simulink model "MX_Gateway"  (Simulink 9.0) (continued)
  CD_FAST_TRAVEL_FUNCTION = Bobcat.Parameter; CreateAliasCD( CD_FAST_TRAVEL_FUNCTION                              ,uint8(255)                 ,'ConstantDataPointer' ,'CONSTANT_DATA' ,'FAST_TRAVEL_FUNCTION')    [cdata.m]    (also in hc12_cdata.m; m4_cdata.m)
  CD_FAST_TRAVEL_THROTTLE_DELAY = Bobcat.Parameter; CreateAliasCD( CD_FAST_TRAVEL_THROTTLE_DELAY                        ,uint16(10)                 ,'ConstantDataPointer' ,'CONSTANT_DATA' ,'FAST_TRAVEL_THROTTLE_DELAY')    [cdata.m]    (also in hc12_cdata.m; m4_cdata.m)
  CD_FAST_TRAVEL_THROTTLE_LIMIT = Bobcat.Parameter; CreateAliasCD( CD_FAST_TRAVEL_THROTTLE_LIMIT                        ,uint16(1000)               ,'ConstantDataPointer' ,'CONSTANT_DATA' ,'FAST_TRAVEL_THROTTLE_LIMIT')    [cdata.m]    (also in hc12_cdata.m; m4_cdata.m)
  CD_FLYWHEEL_TEETH = Bobcat.Parameter; CreateAliasCD( CD_FLYWHEEL_TEETH                                    ,uint8(83)                  ,'ConstantDataPointer' ,'CONSTANT_DATA' ,'FLYWHEEL_TEETH')    [cdata.m]    (also in hc12_cdata.m; m4_cdata.m)
  CD_FUEL_LEVEL_FILTER_FAST_VALUE = Bobcat.Parameter; CreateAliasCD( CD_FUEL_LEVEL_FILTER_FAST_VALUE                      ,uint8(5)                   ,'ConstantDataPointer' ,'CONSTANT_DATA' ,'FUEL_LEVEL_FILTER_FAST_VALUE')    [cdata.m]    (also in hc12_cdata.m; m4_cdata.m)
  CD_FUEL_LEVEL_FILTER_SLOW_VALUE = Bobcat.Parameter; CreateAliasCD( CD_FUEL_LEVEL_FILTER_SLOW_VALUE                      ,uint8(10)                  ,'ConstantDataPointer' ,'CONSTANT_DATA' ,'FUEL_LEVEL_FILTER_SLOW_VALUE')    [cdata.m]    (also in hc12_cdata.m; m4_cdata.m)
  CD_FUEL_PRIMARY_FEEDBACK_FUNCTION = Bobcat.Parameter; CreateAliasCD( CD_FUEL_PRIMARY_FEEDBACK_FUNCTION                    ,uint8(0)                   ,'ConstantDataPointer' ,'CONSTANT_DATA' ,'FUEL_PRIMARY_FEEDBACK_FUNCTION')    [cdata.m]    (also in hc12_cdata.m; m4_cdata.m)
  ... (+246 more)
undefined symbols (not defined in the file or in its folder): sigbuilder_block
undefined symbols (not defined in the file): CD_FUEL_RESOLUTION_TIMER
libraries: simulink x186, MX_Library x107
(part 9 of 48)

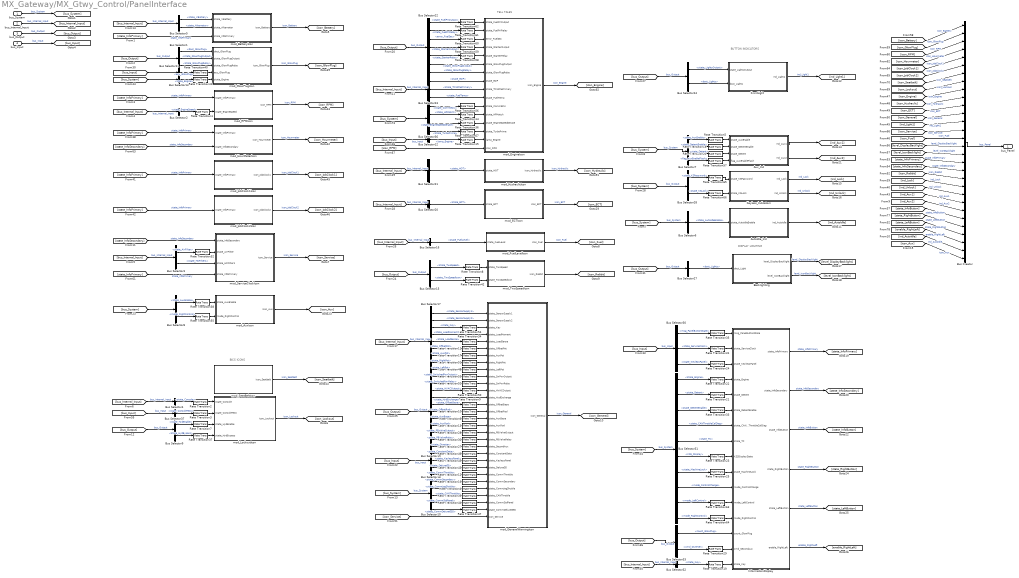
== system MX_Gateway/MX_Gtwy_Control/PanelInterface ==
  note: DISPLAY LIGHTING
  note: BUTTON INDICATORS
  note: TELL TALES
  note: BICS ICONS
  bus_System : Inport
  bus_Internal_Input : Inport  Port=2
  bus_Output : Inport  Port=3
  bus_Input : Inport  Port=4
  AutoIdle_Ind : Subsystem  TreatAsAtomicUnit=on  SystemSampleTime=srate100  RTWFileName=mod_Panel
      settings: RTWSystemCode=Nonreusable function  RTWFileNameOpts=User specified
  Aux_Ind : Subsystem  TreatAsAtomicUnit=on  SystemSampleTime=srate100  RTWFileName=mod_Panel
      settings: RTWSystemCode=Nonreusable function  RTWFileNameOpts=User specified
  Backlighting : Subsystem  TreatAsAtomicUnit=on  SystemSampleTime=srate100  RTWFileName=mod_Panel
      settings: RTWSystemCode=Nonreusable function  RTWFileNameOpts=User specified
  Bus Creator : BusCreator  Inputs=30
  Bus Selector1 : BusSelector  OutputSignals=dout_GlowPlug,state_GlowPlugOutput,state_GlowPlugRelay
  Bus Selector10 : BusSelector  OutputSignals=state_CommThrottle,state_CommSecondary,state_CommJogShuttle,state_CANThrottle,state_CommStdPanel,state_CommDeluxeG55
  Bus Selector11 : BusSelector  OutputSignals=bus_CAN.bus_ECU.ecu_GlowPlug
  Bus Selector12 : BusSelector  OutputSignals=bus_CAN.bus_ECU.lamp_Engine
  Bus Selector13 : BusSelector  OutputSignals=state_Engine
  Bus Selector15 : BusSelector  OutputSignals=state_TwoSpeed,state_TwoSpeedIcon
  Bus Selector16 : BusSelector  OutputSignals=state_FuelLevel
  Bus Selector17 : BusSelector  OutputSignals=state_SensorSupply1,state_SensorSupply2,state_Key,state_LoadMoment,state_LoadSense,state_OffsetPot,state_AuxPot,state_RightPot,state_LeftPot
  Bus Selector18 : BusSelector  OutputSignals=bus_Controller.state_ConstantData,bus_CAN.bus_Keypad.state_KeylessPanel,bus_CAN.bus_DeluxeG5.state_DeluxeG5
  Bus Selector19 : BusSelector  OutputSignals=state_SwitchedPwrOutput,state_SwitchedPwrRelay,state_HVACOutput,state_HydExchange,state_OffsetBase,state_OffsetRod,state_AuxBase,state_AuxRod,state_PBValveOutput,state_PBValveRelay,state_Diverter
  Bus Selector2 : BusSelector  OutputSignals=state_EngineSpeed
  Bus Selector20 : BusSelector  OutputSignals=state_ECT
  Bus Selector21 : BusSelector  OutputSignals=state_HOT
  Bus Selector22 : BusSelector  OutputSignals=state_FuelPriOutput,state_FuelPriRelay,error_FuelSec,state_StarterOutput,state_StarterRelay,state_GlowPlugOutput,state_GlowPlugRelay
  Bus Selector23 : BusSelector  OutputSignals=state_EOP,state_ThrottlePrimary,state_FuelTemp
  Bus Selector24 : BusSelector  OutputSignals=state_LightsOutput,dout_Lights
  Bus Selector27 : BusSelector  OutputSignals=dout_Lights
  Bus Selector28 : BusSelector  OutputSignals=state_KSPassword,state_KSLock
  Bus Selector3 : BusSelector  OutputSignals=state_AirFilter,state_HCFilter1
  Bus Selector30 : BusSelector  OutputSignals=bus_CAN.bus_StdDisplay.msg_PanelButtonState,bus_Controller.state_ServiceClock,bus_CAN.bus_Keypad.state_KeylessPanel
  Bus Selector31 : BusSelector  OutputSignals=state_Engine,state_Detent,state_DetentEnable,state_CANThrottleCalDiag,state_TC,KSS_Display,state_MachineLock,mode_ControlChange,mode_LeftControl,mode_RightControl
  Bus Selector32 : BusSelector  OutputSignals=state_Key
  Bus Selector33 : BusSelector  OutputSignals=count_GlowPlug,cmd_Diverter
  Bus Selector36 : BusSelector  OutputSignals=state_CommECU,state_HPMatch,state_EngineSpeedDerate,state_TurboPrime
  Bus Selector37 : BusSelector  OutputSignals=bus_Controller.state_ConsoleTest
  Bus Selector4 : BusSelector  OutputSignals=state_Console
  Bus Selector5 : BusSelector  OutputSignals=state_VBattery,state_Alternator
  Bus Selector6 : BusSelector  OutputSignals=state_AutoIdleEnable
  Bus Selector7 : BusSelector  OutputSignals=state_AuxEnable,state_DetentEnable,state_Detent,flag_AuxEnableFlash
  Bus Selector8 : BusSelector  OutputSignals=state_AuxEnable,mode_RightControl
  Bus Selector9 : BusSelector  OutputSignals=state_HydEnable,state_HydBypass
  FrontLight : Subsystem  TreatAsAtomicUnit=on  SystemSampleTime=srate100  RTWFileName=mod_Panel
      settings: RTWSystemCode=Nonreusable function  RTWFileNameOpts=User specified
  InformationDisplay : Subsystem  TreatAsAtomicUnit=on  SystemSampleTime=srate100  RTWFileName=mod_Panel
      settings: RTWSystemCode=Nonreusable function  RTWFileNameOpts=User specified
  Keyless_Indicators : Subsystem  TreatAsAtomicUnit=on  SystemSampleTime=srate100  RTWFileName=mod_Panel
      settings: RTWSystemCode=Nonreusable function  RTWFileNameOpts=User specified
  Rate Transition{1..25,27..48,50..67} (65 alike) : RateTransition  Integrity=off
  mod_AuxIcon : Subsystem  TreatAsAtomicUnit=on  SystemSampleTime=srate100  RTWFileName=mod_Panel
      settings: RTWSystemCode=Nonreusable function  RTWFileNameOpts=User specified
  mod_BatteryIcon : Subsystem  TreatAsAtomicUnit=on  SystemSampleTime=srate100  RTWFileName=mod_Panel
      settings: RTWSystemCode=Nonreusable function  RTWFileNameOpts=User specified
  mod_ECTIcon : Subsystem  TreatAsAtomicUnit=on  SystemSampleTime=srate100  RTWFileName=mod_Panel
      settings: RTWSystemCode=Nonreusable function  RTWFileNameOpts=User specified
  mod_EngineIcon : Subsystem  TreatAsAtomicUnit=on  SystemSampleTime=srate100  RTWFileName=mod_Panel
      settings: RTWSystemCode=Nonreusable function  RTWFileNameOpts=User specified
  mod_FuelLevelIcon : Subsystem  TreatAsAtomicUnit=on  SystemSampleTime=srate100  RTWFileName=mod_Panel
      settings: RTWSystemCode=Nonreusable function  RTWFileNameOpts=User specified
  mod_GeneralWarningIcon : Subsystem  TreatAsAtomicUnit=on  SystemSampleTime=srate100  RTWFileName=mod_Panel
      settings: RTWSystemCode=Nonreusable function  RTWFileNameOpts=User specified
  mod_GlowPlugIcon : Subsystem  TreatAsAtomicUnit=on  SystemSampleTime=srate100  RTWFileName=mod_Panel
      settings: RTWSystemCode=Nonreusable function  RTWFileNameOpts=User specified
  mod_HourMeterIcon : Subsystem  TreatAsAtomicUnit=on  SystemSampleTime=srate100  RTWFileName=mod_Panel
      settings: RTWSystemCode=Nonreusable function  RTWFileNameOpts=User specified
  mod_HydraulicIcon : Subsystem  TreatAsAtomicUnit=on  SystemSampleTime=srate100  RTWFileName=mod_Panel
      settings: RTWSystemCode=Nonreusable function  RTWFileNameOpts=User specified
  mod_JobClock1Icon : Subsystem  TreatAsAtomicUnit=on  SystemSampleTime=srate100  RTWFileName=mod_Panel
      settings: RTWSystemCode=Nonreusable function  RTWFileNameOpts=User specified
  mod_JobClock2Icon : Subsystem  TreatAsAtomicUnit=on  SystemSampleTime=srate100  RTWFileName=mod_Panel
      settings: RTWSystemCode=Nonreusable function  RTWFileNameOpts=User specified
  mod_LockoutIcon : Subsystem  TreatAsAtomicUnit=on  SystemSampleTime=srate100  RTWFileName=mod_Panel
      settings: RTWSystemCode=Nonreusable function  RTWFileNameOpts=User specified
  mod_RPMIcon : Subsystem  TreatAsAtomicUnit=on  SystemSampleTime=srate100  RTWFileName=mod_Panel
      settings: RTWSystemCode=Nonreusable function  RTWFileNameOpts=User specified
  mod_SeatBeltIcon : Subsystem  TreatAsAtomicUnit=on  SystemSampleTime=srate100  RTWFileName=mod_Panel
      settings: RTWSystemCode=Nonreusable function  RTWFileNameOpts=User specified
  mod_ServiceClockIcon : Subsystem  TreatAsAtomicUnit=on  SystemSampleTime=srate100  RTWFileName=mod_Panel
      settings: RTWSystemCode=Nonreusable function  RTWFileNameOpts=User specified
  mod_TwoSpeedIcon : Subsystem  TreatAsAtomicUnit=on  SystemSampleTime=srate100  RTWFileName=mod_Panel
      settings: RTWSystemCode=Nonreusable function  RTWFileNameOpts=User specified
  bus_Panel : Outport
  wires:
  Bus Selector32/1 -> Rate Transition19/1  "<state_Key>"
  Bus Selector33/1 -> InformationDisplay/14(count_GlowPlug)  "<count_GlowPlug>"
  Bus Selector30/2 -> Rate Transition31/1  "<state_ServiceClock>"
  Bus Selector30/1 -> Rate Transition35/1  "<msg_PanelButtonState>"
  [bus_Output] -> Bus Selector33/1  "bus_Output"
  [bus_Internal_Input] -> Bus Selector32/1  "bus_Internal_Input"
  [bus_System] -> Bus Selector31/1  "bus_System"
  [bus_Input] -> Bus Selector30/1  "bus_Input"
  InformationDisplay/3(state_InfoButton) -> [state_InfoButton]  "state_InfoButton"
  InformationDisplay/2(state_InfoSecondary) -> [state_InfoSecondary]  "state_InfoSecondary"
  InformationDisplay/1(state_InfoPrimary) -> [state_InfoPrimary]  "state_InfoPrimary"
  Bus Selector28/2 -> Rate Transition66/1  "<state_KSLock>"
  Bus Selector28/1 -> Rate Transition65/1  "<state_KSPassword>"
  [bus_System] -> Bus Selector28/1  "bus_Output"
  Bus Selector27/1 -> Backlighting/1(dout_Light)  "<dout_Lights>"
  [bus_Output] -> Bus Selector27/1  "bus_Output"
  Bus Selector24/2 -> FrontLight/2(dout_Lights)  "<dout_Lights>"
  Bus Selector24/1 -> FrontLight/1(state_LightsOutput)  "<state_LightsOutput>"
  [bus_Output] -> Bus Selector24/1  "bus_Output"
  Aux_Ind/1(ind_Aux1) -> [ind_Aux1]
  Keyless_Indicators/2(ind_Unlock) -> [ind_Unlock]  "ind_Unlock"
  Keyless_Indicators/1(ind_Lock) -> [ind_Lock]  "ind_Lock"
  Backlighting/2(level_IconBacklight) -> [level_IconBacklight]  "level_IconBacklight"
  Backlighting/1(level_DisplayBacklight) -> [level_DisplayBacklight]  "level_DisplayBacklight"
  FrontLight/1(ind_Light1) -> [ind_Light1]  "ind_Light1"
  Bus Selector23/1 -> mod_EngineIcon/8(state_EOP)  "<state_EOP>"
  [bus_Internal_Input] -> Bus Selector23/1  "bus_Internal_Input"
  Bus Selector22/5 -> Rate Transition58/1  "<state_StarterRelay>"
  Bus Selector22/4 -> Rate Transition59/1  "<state_StarterOutput>"
  Bus Selector22/3 -> Rate Transition60/1  "<error_FuelSec>"
  Bus Selector22/2 -> Rate Transition61/1  "<state_FuelPriRelay>"
  Bus Selector22/1 -> Rate Transition62/1  "<state_FuelPriOutput>"
  [bus_Output] -> Bus Selector22/1  "bus_Output"
  Bus Selector21/1 -> mod_HydraulicIcon/1(state_HOT)  "<state_HOT>"
  [bus_Internal_Input] -> Bus Selector21/1  "bus_Internal_Input"
  Bus Selector20/1 -> mod_ECTIcon/1(state_ECT)  "<state_ECT>"
  [bus_Internal_Input] -> Bus Selector20/1  "bus_Internal_Input"
  Bus Selector19/2 -> Rate Transition52/1  "<state_SwitchedPwrRelay>"
  Bus Selector19/1 -> Rate Transition53/1  "<state_SwitchedPwrOutput>"
  Bus Selector17/3 -> Rate Transition54/1  "<state_Key>"
  Bus Selector17/2 -> mod_GeneralWarningIcon/2(state_SensorSupply2)  "<state_SensorSupply2>"
  Bus Selector17/1 -> mod_GeneralWarningIcon/1(state_SensorSupply1)  "<state_SensorSupply1>"
  [bus_Output] -> Bus Selector19/1  "bus_Output"
  [bus_Internal_Input] -> Bus Selector17/1  "bus_Internal_Input"
  Bus Selector18/2 -> Rate Transition45/1  "<state_KeylessPanel>"
  Bus Selector18/1 -> Rate Transition46/1  "<state_ConstantData>"
  Bus Selector16/1 -> mod_FuelLevelIcon/1(state_FuelLevel)  "<state_FuelLevel>"
  Bus Selector15/2 -> Rate Transition42/1  "<state_TwoSpeedIcon>"
  [bus_Internal_Input] -> Bus Selector16/1  "bus_Internal_Input"
  [bus_Output] -> Bus Selector15/1  "bus_Output"
  [bus_Input] -> Bus Selector18/1  "bus_Input"
  mod_TwoSpeedIcon/1(icon_Rabbit) -> [icon_Rabbit]
  mod_FuelLevelIcon/1(icon_Fuel) -> [icon_Fuel]  "icon_Fuel"
  mod_GeneralWarningIcon/1(icon_General) -> [icon_General]  "icon_General"
  mod_ECTIcon/1(icon_ECT) -> [icon_ECT]  "icon_ECT"
  mod_EngineIcon/1(icon_Engine) -> [icon_Engine]  "icon_Engine"
  mod_HydraulicIcon/1(icon_Hydraulic) -> [icon_Hydraulic]  "icon_Hydraulic"
  Bus Selector4/1 -> Rate Transition2/1  "<state_Console>"
  [bus_Internal_Input] -> Bus Selector4/1  "bus_Internal_Input"
  Bus Selector3/2 -> mod_ServiceClockIcon/3(state_HCFilter1)  "<state_HCFilter1>"
  Bus Selector3/1 -> Rate Transition32/1  "<state_AirFilter>"
  [bus_Internal_Input] -> Bus Selector3/1  "bus_Internal_Input"
  [state_InfoPrimary] -> mod_ServiceClockIcon/4(state_InfoPrimary)  "state_InfoPrimary"
  mod_ServiceClockIcon/1(icon_Service) -> [icon_Service]  "icon_Service"
  [state_InfoSecondary] -> mod_ServiceClockIcon/1(state_InfoSecondary)  "state_InfoSecondary"
  mod_SeatBeltIcon/1(icon_Seatbelt) -> [icon_Seatbelt]  "icon_Seatbelt"
  mod_LockoutIcon/1(icon_Lockout) -> [icon_Lockout]  "icon_Lockout"
  Bus Selector2/1 -> Rate Transition1/1  "<state_EngineSpeed>"
  [bus_Internal_Input] -> Bus Selector2/1  "bus_Internal_Input"
  [state_InfoPrimary] -> mod_RPMIcon/1(state_InfoPrimary)  "state_InfoPrimary"
  Bus Selector1/3 -> mod_GlowPlugIcon/3(state_GlowPlugRelay)  "<state_GlowPlugRelay>"
  Bus Selector1/2 -> mod_GlowPlugIcon/2(state_GlowPlugOutput)  "<state_GlowPlugOutput>"
  Bus Selector1/1 -> mod_GlowPlugIcon/1(dout_GlowPlug)  "<dout_GlowPlug>"
  [bus_Output] -> Bus Selector1/1  "bus_Output"
  [state_InfoSecondary] -> mod_HourMeterIcon/2(state_InfoSecondary)  "state_InfoSecondary"
  mod_JobClock2Icon/1(icon_JobClock2) -> [icon_JobClock2]  "icon_JobClock2"
  [state_InfoPrimary] -> mod_JobClock2Icon/1(state_InfoPrimary)  "state_InfoPrimary"
  mod_JobClock1Icon/1(icon_JobClock1) -> [icon_JobClock1]  "icon_JobClock1"
  [state_InfoPrimary] -> mod_JobClock1Icon/1(state_InfoPrimary)  "state_InfoPrimary"
  mod_RPMIcon/1(icon_RPM) -> [icon_RPM]  "icon_RPM"
  mod_HourMeterIcon/1(icon_Hourmeter) -> [icon_Hourmeter]  "icon_Hourmeter"
  [state_InfoPrimary] -> mod_HourMeterIcon/1(state_InfoPrimary)  "state_InfoPrimary"
  mod_GlowPlugIcon/1(icon_GlowPlug) -> [icon_GlowPlug]  "icon_GlowPlug"
  mod_BatteryIcon/1(icon_Battery) -> [icon_Battery]  "icon_Battery"
  [state_InfoPrimary] -> mod_BatteryIcon/3(state_InfoPrimary)  "state_InfoPrimary"
  Bus Selector5/2 -> mod_BatteryIcon/2(state_Alternator)  "<state_Alternator>"
  Bus Selector5/1 -> mod_BatteryIcon/1(state_VBattery)  "<state_VBattery>"
  [bus_Internal_Input] -> Bus Selector5/1  "bus_Internal_Input"
  [icon_Battery] -> Bus Creator/1  "icon_Battery"
  [icon_GlowPlug] -> Bus Creator/2  "icon_GlowPlug"
  [icon_RPM] -> Bus Creator/3  "icon_RPM"
  [icon_Hourmeter] -> Bus Creator/4  "icon_Hourmeter"
  [icon_JobClock1] -> Bus Creator/5  "icon_JobClock1"
  [icon_JobClock2] -> Bus Creator/6  "icon_JobClock2"
  [icon_Seatbelt] -> Bus Creator/7  "icon_Seatbelt"
  [icon_Lockout] -> Bus Creator/8  "icon_Lockout"
  [icon_Engine] -> Bus Creator/9  "icon_Engine"
  [icon_Hydraulic] -> Bus Creator/10  "icon_Hydraulic"
  [icon_ECT] -> Bus Creator/11  "icon_ECT"
  [icon_General] -> Bus Creator/12  "icon_General"
  [ind_Light1] -> Bus Creator/13  "ind_Light1"
  [icon_Service] -> Bus Creator/14  "icon_Service"
  [icon_Fuel] -> Bus Creator/15  "icon_Fuel"
  [level_DisplayBacklight] -> Bus Creator/16  "level_DisplayBacklight"
  [level_IconBacklight] -> Bus Creator/17  "level_IconBacklight"
  [state_InfoPrimary] -> Bus Creator/18  "state_InfoPrimary"
  [state_InfoSecondary] -> Bus Creator/19  "state_InfoSecondary"
  bus_System/1 -> [bus_System]  "bus_System"
  bus_Internal_Input/1 -> [bus_Internal_Input]  "bus_Internal_Input"
  bus_Output/1 -> [bus_Output]  "bus_Output"
  bus_Input/1 -> [bus_Input]  "bus_Input"
  Bus Creator/1 -> bus_Panel/1  "bus_Panel"
  Bus Selector18/3 -> Rate Transition43/1  "<state_DeluxeG5>"
  Bus Selector23/2 -> mod_EngineIcon/9(state_ThrottlePrimary)  "<state_ThrottlePrimary>"
  [bus_System] -> Bus Selector36/1
  Bus Selector36/1 -> Rate Transition56/1  "<state_CommECU>"
  [ind_Lock] -> Bus Creator/21  "ind_Lock"
  [ind_Unlock] -> Bus Creator/22  "ind_Unlock"
  [ind_Aux1] -> Bus Creator/23  "ind_Aux1"
  [state_InfoButton] -> Bus Creator/25  "state_InfoButton"
  Bus Selector37/1 -> Rate Transition3/1  "<state_ConsoleTest>"
  [bus_Input] -> Bus Selector37/1  "bus_Input"
  Bus Selector19/3 -> Rate Transition50/1  "<state_HVACOutput>"
  Rate Transition1/1 -> mod_RPMIcon/2(state_EngineSpeed)
  Rate Transition3/1 -> mod_LockoutIcon/2(state_ConsoleTest)
  Rate Transition19/1 -> InformationDisplay/16(state_Key)
  Rate Transition22/1 -> InformationDisplay/4(state_Engine)
  Rate Transition31/1 -> InformationDisplay/2(state_ServiceClock)
  Rate Transition35/1 -> InformationDisplay/1(msg_PanelButtonState)
  Rate Transition42/1 -> mod_TwoSpeedIcon/2(state_TwoSpeedIcon)
  Rate Transition50/1 -> mod_GeneralWarningIcon/12(state_HVACOutput)
  Rate Transition52/1 -> mod_GeneralWarningIcon/11(state_SwPwrRelay)
  Rate Transition53/1 -> mod_GeneralWarningIcon/10(state_SwPwrOutput)
  Rate Transition54/1 -> mod_GeneralWarningIcon/3(state_Key)
  Rate Transition58/1 -> mod_EngineIcon/5(state_StarterRelay)
  Rate Transition59/1 -> mod_EngineIcon/4(state_StarterOutput)
  Rate Transition60/1 -> mod_EngineIcon/3(error_FuelSec)
  Rate Transition61/1 -> mod_EngineIcon/2(state_FuelPriRelay)
  Rate Transition62/1 -> mod_EngineIcon/1(state_FuelPriOutput)
  Rate Transition65/1 -> Keyless_Indicators/1(state_KSPassword)
  Rate Transition66/1 -> Keyless_Indicators/2(state_KSLock)
  InformationDisplay/5(state_LeftButton) -> [state_LeftButton]  "state_LeftButton"
  InformationDisplay/4(state_RightButton) -> [state_RightButton]  "state_RightButton"
  [state_RightButton] -> Bus Creator/26  "state_LeftButton"
  [state_LeftButton] -> Bus Creator/27  "state_RightButton"
  InformationDisplay/6(enable_RightLeft) -> [enable_RightLeft]  "enable_RightLeft"
  [enable_RightLeft] -> Bus Creator/28  "enable_RightLeft"
  [icon_Rabbit] -> Bus Creator/20  "icon_Rabbit"
  AutoIdle_Ind/1(ind_AutoIdle) -> [ind_AutoIdle]
  [bus_System] -> Bus Selector6/1  "bus_System"
  Bus Selector6/1 -> AutoIdle_Ind/1(state_AutoIdleEnable)  "<state_AutoIdleEnable>"
  [bus_System] -> Bus Selector7/1  "bus_System"
  Bus Selector7/1 -> Rate Transition5/1  "<state_AuxEnable>"
  [ind_AutoIdle] -> Bus Creator/29  "ind_AutoIdle"
  Rate Transition5/1 -> Aux_Ind/1(state_AuxEnable)
  [bus_Output] -> Bus Selector9/1  "bus_Output"
  Bus Selector9/1 -> Rate Transition7/1  "<state_HydEnable>"
  Rate Transition2/1 -> mod_LockoutIcon/1(state_Console)
  Rate Transition7/1 -> mod_LockoutIcon/3(state_HydEnable)
  Rate Transition8/1 -> mod_TwoSpeedIcon/1(state_TwoSpeed)
  Bus Selector15/1 -> Rate Transition8/1  "<state_TwoSpeed>"
  Bus Selector17/5 -> Rate Transition34/1  "<state_LoadSense>"
  Bus Selector17/6 -> Rate Transition11/1  "<state_OffsetPot>"
  Bus Selector19/4 -> mod_GeneralWarningIcon/13(state_HydExchange)  "<state_HydExchange>"
  Bus Selector19/5 -> Rate Transition9/1  "<state_OffsetBase>"
  Bus Selector19/6 -> Rate Transition10/1  "<state_OffsetRod>"
  Rate Transition9/1 -> mod_GeneralWarningIcon/14(state_OffsetBase)
  Rate Transition10/1 -> mod_GeneralWarningIcon/15(state_OffsetRod)
  [bus_System] -> Bus Selector10/1  "bus_System"
  Bus Selector10/1 -> Rate Transition12/1  "<state_CommThrottle>"
  [bus_Input] -> Bus Selector12/1  "bus_Input"
  Bus Selector12/1 -> Rate Transition15/1  "<lamp_Engine>"
  Bus Selector10/2 -> Rate Transition16/1  "<state_CommSecondary>"
  Bus Selector10/3 -> Rate Transition4/1  "<state_CommJogShuttle>"
  Bus Selector19/7 -> Rate Transition6/1  "<state_AuxBase>"
  Bus Selector19/8 -> Rate Transition13/1  "<state_AuxRod>"
  Rate Transition6/1 -> mod_GeneralWarningIcon/16(state_AuxBase)
  Rate Transition13/1 -> mod_GeneralWarningIcon/17(state_AuxRod)
  Bus Selector17/7 -> Rate Transition17/1  "<state_AuxPot>"
  Bus Selector7/2 -> Rate Transition18/1  "<state_DetentEnable>"
  Rate Transition18/1 -> Aux_Ind/2(state_DetentEnable)
  Aux_Ind/2(ind_Aux2) -> [ind_Aux2]
  [ind_Aux2] -> Bus Creator/24  "ind_Aux2"
  Bus Selector7/3 -> Rate Transition20/1  "<state_Detent>"
  Rate Transition20/1 -> Aux_Ind/3(state_Detent)
  Rate Transition21/1 -> InformationDisplay/5(state_Detent)
  Rate Transition15/1 -> mod_EngineIcon/15(lamp_Engine)
  Rate Transition56/1 -> mod_EngineIcon/11(state_CommECU)
  Rate Transition33/1 -> InformationDisplay/6(state_DetentEnable)
  Bus Selector36/3 -> Rate Transition36/1  "<state_EngineSpeedDerate>"
  Rate Transition36/1 -> mod_EngineIcon/13(state_EngineSpeedDerate)
  mod_AuxIcon/1(icon_Aux) -> [icon_Aux]
  [bus_System] -> Bus Selector8/1
  Bus Selector8/1 -> Rate Transition38/1  "<state_AuxEnable>"
  [icon_Aux] -> Bus Creator/30  "icon_Aux"
  Rate Transition38/1 -> mod_AuxIcon/1(state_AuxEnable)
  Bus Selector9/2 -> Rate Transition37/1  "<state_HydBypass>"
  Rate Transition37/1 -> mod_LockoutIcon/4(state_HydBypass)
  Bus Selector11/1 -> Rate Transition40/1  "<ecu_GlowPlug>"
  [bus_Input] -> Bus Selector11/1
  Rate Transition40/1 -> mod_GlowPlugIcon/4(ecu_GlowPlug)
  [bus_System] -> Bus Selector13/1
  Bus Selector13/1 -> Rate Transition41/1  "<state_Engine>"
  Rate Transition41/1 -> mod_GlowPlugIcon/5(state_Engine)
  [icon_RPM] -> mod_EngineIcon/16(icon_RPM)
  Bus Selector22/6 -> mod_EngineIcon/6(state_GlowPlugOutput)  "<state_GlowPlugOutput>"
  Bus Selector22/7 -> mod_EngineIcon/7(state_GlowPlugRelay)  "<state_GlowPlugRelay>"
  Bus Selector36/2 -> Rate Transition14/1  "<state_HPMatch>"
  Rate Transition14/1 -> mod_EngineIcon/12(state_HPMatch)
  Bus Selector23/3 -> mod_EngineIcon/10(state_FuelTemp)  "<state_FuelTemp>"
  Bus Selector19/9 -> Rate Transition28/1  "<state_PBValveOutput>"
  Bus Selector19/10 -> Rate Transition30/1  "<state_PBValveRelay>"
  Bus Selector31/6 -> Rate Transition23/1  "<KSS_Display>"
  Bus Selector31/1 -> Rate Transition22/1  "<state_Engine>"
  Bus Selector31/2 -> Rate Transition21/1  "<state_Detent>"
  Bus Selector31/3 -> Rate Transition33/1  "<state_DetentEnable>"
  Bus Selector30/3 -> Rate Transition24/1  "<state_KeylessPanel>"
  Rate Transition23/1 -> InformationDisplay/9(KSSDisplayState)
  Rate Transition24/1 -> InformationDisplay/3(state_KeylessPanel)
  Rate Transition25/1 -> InformationDisplay/10(state_MachineLock)
  Bus Selector31/7 -> Rate Transition25/1  "<state_MachineLock>"
  Bus Selector33/2 -> Rate Transition29/1  "<cmd_Diverter>"
  Rate Transition29/1 -> InformationDisplay/15(cmd_SecondAux)
  Rate Transition32/1 -> mod_ServiceClockIcon/2(state_AirFilter)
  Rate Transition28/1 -> mod_GeneralWarningIcon/18(state_PBValveOutput)
  Rate Transition30/1 -> mod_GeneralWarningIcon/19(state_PBValveRelay)
  Rate Transition16/1 -> mod_GeneralWarningIcon/25(state_CommSecondary)
  Rate Transition12/1 -> mod_GeneralWarningIcon/24(state_CommThrottle)
  Rate Transition45/1 -> mod_GeneralWarningIcon/22(state_KeylessPanel)
  Rate Transition43/1 -> mod_GeneralWarningIcon/23(state_DeluxeG5)
  Rate Transition46/1 -> mod_GeneralWarningIcon/21(state_ConstantData)
  Bus Selector10/4 -> Rate Transition39/1  "<state_CANThrottle>"
  Bus Selector10/5 -> Rate Transition44/1  "<state_CommStdPanel>"
  Bus Selector10/6 -> Rate Transition47/1  "<state_CommDeluxeG55>"
  Rate Transition4/1 -> mod_GeneralWarningIcon/26(state_CommJogShuttle)
  Rate Transition39/1 -> mod_GeneralWarningIcon/27(state_CANThrottle)
  Rate Transition44/1 -> mod_GeneralWarningIcon/28(state_CommStdPanel)
  Rate Transition47/1 -> mod_GeneralWarningIcon/29(state_CommDeluxeG55)
  [icon_Service] -> mod_GeneralWarningIcon/30(icon_Service)
  Bus Selector31/5 -> InformationDisplay/8(state_TC)  "<state_TC>"
  Bus Selector31/4 -> InformationDisplay/7(state_CANThrottleCalDiag)  "<state_CANThrottleCalDiag>"
  Rate Transition27/1 -> mod_GeneralWarningIcon/20(state_SecondAux)
  Bus Selector19/11 -> Rate Transition27/1  "<state_Diverter>"
  Bus Selector31/8 -> InformationDisplay/11(mode_ControlChange)  "<mode_ControlChange>"
  Bus Selector17/8 -> Rate Transition51/1  "<state_RightPot>"
  Bus Selector17/9 -> Rate Transition48/1  "<state_LeftPot>"
  Bus Selector8/2 -> Rate Transition55/1  "<mode_RightControl>"
  Rate Transition55/1 -> mod_AuxIcon/2(mode_RightControl)
  Bus Selector7/4 -> Rate Transition57/1  "<flag_AuxEnableFlash>"
  Rate Transition57/1 -> Aux_Ind/4(flag_AuxEnableFlash)
  Bus Selector31/9 -> Rate Transition63/1  "<mode_LeftControl>"
  Bus Selector31/10 -> Rate Transition64/1  "<mode_RightControl>"
  Rate Transition63/1 -> InformationDisplay/12(mode_LeftControl)
  Rate Transition64/1 -> InformationDisplay/13(mode_RightControl)
  Rate Transition67/1 -> mod_EngineIcon/14(state_TurboPrime)
  Bus Selector36/4 -> Rate Transition67/1  "<state_TurboPrime>"
  Bus Selector17/4 -> mod_GeneralWarningIcon/4(state_LoadMoment)  "<state_LoadMoment>"
  Rate Transition34/1 -> mod_GeneralWarningIcon/5(state_LoadSense)
  Rate Transition11/1 -> mod_GeneralWarningIcon/6(state_OffsetPot)
  Rate Transition17/1 -> mod_GeneralWarningIcon/7(state_AuxPot)
  Rate Transition51/1 -> mod_GeneralWarningIcon/8(state_RightPot)
  Rate Transition48/1 -> mod_GeneralWarningIcon/9(state_LeftPot)
